simulink model "PlanarModelandControl"  (R2026a)
settings: Solver=FixedStepDiscrete  StopTime=StopTime  FixedStep=Ts  MaxStep=0  RelTol=1e-5  AbsTol=1e-3
PostLoadFcn:
  | Controles_Ativos = 1;
  | Vx_Ref = 0;
  | Vx_init = 0;
  | t_default = [0; 10000];
  | Brake_Cmd = [t_default, [0; 0]];
  | Accel_Cmd = [t_default, [0; 0]];
  | Steer_Cmd = [t_default, [0; 0]];
  | Flag_CruiseControl = 0;
  | Ts = 0.001;
  | load('TC_LUT.mat')
InitFcn:
  | % Carrega parâmetros gerais (Massa, Ganhos PID, Geometria)
  | run('Parameters.m');
  | 
  | % Carrega as Tabelas (Lookup Tables)
  | run('curva_steering.m');
  | run('curva_tq_motor.m');
  | load('TC_LUT.mat')
model workspace (MAT data in the model file):
  A = 1.22
  Ack = 1
  Cd = 1.1
  Cl = -2
  Cs = [19075.58 25992.8 31792.64 35898.02 39044.37]
  Fz0x = -811.9083
  Fz0y = -210.9467
  Fz_cs = [222.41 444.82 667.23 889.64 1112.05]
  IAY = 0
  IAx = 0
  IAy = 0
  Im = 0.00033
  It = 0.0094
  Iw = 0.1578
  Iw_ = 0.7
  Iz_car = 180
  K_50_understeer = 0.005184
  K_Stability = 1
  K_oversteer = -0.001
  Kappa_F = 0
  Kappa_R = 0
  Km_FM = 110000
  Km_Fm = 110000
  Km_RM = 120000
  Km_Rm = 120000
  KphiARB_F = 0
  KphiARB_R = 11000
  KphiF = 17054.5
  KphiR = 40034.8
  Kphim_F = 137500
  Kphim_R = 48600
  L = 1.53
  M = 300
  Mgt = 11.72
  P = 68.9476
  P0 = 55.4852
  R = 0.2032
  Rcurva = 20
  Re = 0.2
  SR = 3
  TV_On = 1
  Ts = 0.001
  W = 2940
  Wf = 308.034
  Wr = 339.426
  Ws = 2099.34
  YLL = 1.11022302463e-16
  cx1 = 1.3668
  cy1 = 1.839
  dpi = 0.242630467224
  dx1 = 2.1568
  dx2 = -0.065814
  dx3 = 0.0011254
  dy1 = 2.453
  dy2 = -0.11197
  dy3 = 10.2899
  ex1 = -0.19815
  ex2 = -0.62529
  ex3 = 1.3396
  ex4 = 0.0029464
  ey1 = 0.96085
  ey2 = -0.28662
  ey3 = 0.14389
  ey4 = 4.9274
  ey5 = -70.7165
  g = 9.81
  hcg = 0.252
  hcgs = 0.202
  hrc_f = 0.056
  hrc_r = 0.084
  hs_f = 0.222
  hs_r = 0.222
  hx1 = -0.003859
  hx2 = -0.000374
  hy1 = -0.000403
  hy2 = -0.000261
  kx1 = -36.9039
  kx2 = 22.3857
  kx3 = 0.61844
  ky1 = -83.2526
  ky2 = -5.565
  ky3 = 0.88491
  ky4 = 2.9994
  ky5 = -99.9393
  ky6 = -3.4705
  ky7 = -0.91452
  lf = 0.8415
  lr = 0.6885
  mass_FL = 67.5
  mass_FR = 67.5
  mass_RL = 82.5
  mass_RR = 82.5
  mi = 2
  ms = 240
  pty1 = 0.005744
  pty2 = 19.1334
  px1 = -0.039964
  px2 = -0.15015
  px3 = -0.32528
  px4 = -0.063273
  py1 = 0.38578
  py2 = 1.2048
  py3 = -0.14544
  py4 = 0.092886
  py5 = -0.82901
  r = 9
  rhoar = 1.22
  s_f = 1
  s_r = 0.9
  tf = 1.25
  tr = 1.25
  vx1 = -0.11922
  vx2 = 0.002205
  vy1 = 0.032907
  vy2 = 0.018068
  vy3 = -1.0429
  vy4 = 0.53712
  x_fl = 0.8415
  x_fr = 0.8415
  x_rl = 0.6885
  x_rr = 0.6885
  xs_f = 0.826
  xs_r = 0.704
  y_fl = 0.6
  y_fr = 0.6
  y_rl = 0.59
  y_rr = 0.59
values from neighbouring files (symbols this model uses):
  GG_mode = 0    [autocross.m]    (also in teste.mat)
  Gr = 11.72    [Parameters.m]    (also in teste.mat)
  K_variavel = [-0.001, 0, 0.0008, 0.0012, 0.0015]    [Parameters.m]    (also in teste.mat = [-0.001 0 0.0008 0.0012 0.0015])
  Ki_data = [283117.9493, 114831.615, 51596.0519, 30556.5344, 17525.221, 11773.8301, 10567.5001]*0.008    [Parameters.m]    (also in teste.mat = [2264.9435944 918.65292 412.7684152 244.4522752 140.201768 9)
  Kp_data = [2877.5702, 2303.9999, 1972.0835, 1556.8775, 1258.2451, 1022.7085, 982.7861, ]*5    [Parameters.m]    (also in teste.mat = [14387.851 11519.9995 9860.4175 7784.3875 6291.2255 5113.542)
  Mz_maxF = [0 66.3 132.6 198.9 265.2 330.23 396.52 462.83 529.112 595.42 661.73 728.03 794.33 860.62 926.92 993.23 1058.25 1124.55 1190.85 1257.15 1323.45 1389.75 1456.05 1522.35 1588.65 1654.95]    [Parameters.m]    (also in teste.mat = [0 66.3 132.6 198.9 265.2 330.23 396.52 462.83 529.112 595.4)
  Mz_minF = [0 -66.3 -132.6 -198.9 -265.2 -330.23 -396.52 -462.83 -529.112 -595.42 -661.73 -728.03 -794.33 -860.62 -926.92 -993.23 -1058.25 -1124.55 -1190.85 -1257.15 -1323.45 -1389.75 -1456.05 -1522.35 -1588.65 -1654.95]    [Parameters.m]    (also in teste.mat = [0 -66.3 -132.6 -198.9 -265.2 -330.23 -396.52 -462.83 -529.1)
  pedal = [0 4 8 12 16 20 24 28 32 36 40 44 48 52 56 60 64 68 72 76 80 84 88 92 96 100]    [Parameters.m]    (also in teste.mat = [0 4 8 12 16 20 24 28 32 36 40 44 ... (26 elements, 1x26)])
  rpm_355_novo = [0 10050 10100 10140 10170 10210 10250 10280 10320 10360         10390 10420 10460 10620 10770 10920 11050 11170 11280         11380 11470 11550 11625 11680 11730 11775 11790 11800]    [curva_tq_motor.m]    (also in teste.mat = [0 10050 10100 10140 10170 10210 10250 10280 10320 10360 103)
  Steering_inner = [-29.018 ... (first row only)    [curva_steering.m]    (also in teste.mat = [-29.018 -25.595 -22.357 -19.265 -16.292 -13.415 -10.619 -7.)
  Steering_outer = [-25.111 ... (first row only)    [curva_steering.m]    (also in teste.mat = [-25.111 -22.589 -20.082 -17.585 -15.094 -12.604 -10.11 -7.6)
  steering_vol = [-105 ... (first row only)    [curva_steering.m]    (also in teste.mat = [-105 -94.5 -84 -73.5 -63 -52.5 -42 -31.5 -21 -10.5 0 10.5 .)
  torque_355_novo = [16.2 16.2 16.0 15.8 15.6 15.4 15.2 15.0 14.8 14.6            14.4 14.2 14.0 13.0 12.0 11.0 10.0 9.0 8.0            7.0 6.0 5.0 4.0 3.0 2.0 1.0 0.5 0.0]    [curva_tq_motor.m]    (also in teste.mat = [16.2 16.2 16 15.8 15.6 15.4 15.2 15 14.8 14.6 14.4 14.2 ...)
  Vx = [0, 12.5, 23.6, 30, 35]    [Parameters.m]    (also in teste.mat = [0 12.5 23.6 30 35])
  Vx_axis = [5, 10, 15, 20, 25, 30, 35]    [Parameters.m]    (also in teste.mat = [5 10 15 20 25 30 35])
undefined symbols (not defined in the file or in its folder): DiscIntNeverNeededParam, magnitude
libraries: simulink x5, fuzblock x4, sl_discontinuities x3, simulink_extras x3, slpidlib x1
(part 4 of 4)

== system PlanarModelandControl/Planta/Dinamica do Veículo/Pacejka/Pacejka Long FL /Dx ==
  Dfz : Inport
  Bus Element In1 : Inport  Port=2  PortName=Fz
  Constant : Constant  Value=dx1  SampleTime=0.01
  Gain : Gain  Gain=dx2
  Gain1 : Gain  Gain=1+px1*dpi+px2*dpi^2
  Gain2 : Gain  Gain=1-dx3*IAx^2
  Gain3 : Gain  Gain=-1
  Product : Product
  Sum : Sum  Inputs=|++
  Dx : Outport
  wires:
  Constant/1 -> Sum/1
  Gain/1 -> Sum/2
  Dfz/1 -> Gain/1
  Sum/1 -> Gain1/1
  Product/1 -> Dx/1
  Bus Element In1/1 -> Gain3/1
  Gain1/1 -> Gain2/1
  Gain2/1 -> Product/1
  Gain3/1 -> Product/2

== system PlanarModelandControl/Planta/Dinamica do Veículo/Pacejka/Pacejka Long FL /Ex ==
  Dfz : Inport
  SL : Inport  Port=2
  Abs : Abs  SaturateOnIntegerOverflow=off
  Constant : Constant  Value=ex1  SampleTime=0.01
  Constant2 : Constant  SampleTime=0.01
  Gain : Gain  Gain=ex3
  Gain1 : Gain  Gain=ex4
  Gain2 : Gain  Gain=ex2
  Min : MinMax  Inputs=2
  Product : Product
  Product1 : Product
  Sign : Signum
  Square : Math  Operator=square
  Sum : Sum  Inputs=|+++
  Sum1 : Sum  Inputs=|+-
  Ex : Outport
  wires:
  Constant/1 -> Sum/1
  Gain2/1 -> Sum/2
  Square/1 -> Gain/1
  Gain/1 -> Sum/3
  Sum/1 -> Product/1
  Sum1/1 -> Product/2
  Product/1 -> Min/1
  Sign/1 -> Product1/2
  Abs/1 -> Product1/1
  Constant2/1 -> Min/2, Sum1/1, Abs/1
  Gain1/1 -> Sum1/2
  Product1/1 -> Gain1/1
  Dfz/1 -> Square/1, Gain2/1
  SL/1 -> Sign/1
  Min/1 -> Ex/1

== system PlanarModelandControl/Planta/Dinamica do Veículo/Pacejka/Pacejka Long FL /Kx ==
  Bus Element In1 : Inport  PortName=Fz
  Dfz : Inport  Port=2
  Constant : Constant  Value=kx1  SampleTime=0.01
  Exp : Math
  Gain : Gain  Gain=kx3
  Gain1 : Gain  Gain=kx2
  Gain2 : Gain  Gain=-1
  Gain3 : Gain  Gain=1+px3*dpi+px4*dpi^2
  Product : Product
  Product1 : Product
  Sum : Sum  Inputs=|++
  Kx : Outport
  wires:
  Bus Element In1/1 -> Gain2/1
  Product/1 -> Product1/1
  Exp/1 -> Product1/2
  Gain1/1 -> Sum/2
  Sum/1 -> Product/2
  Product1/1 -> Gain3/1
  Constant/1 -> Sum/1
  Gain/1 -> Exp/1
  Dfz/1 -> Gain1/1, Gain/1
  Gain2/1 -> Product/1
  Gain3/1 -> Kx/1

== system PlanarModelandControl/Planta/Dinamica do Veículo/Pacejka/Pacejka Long FL /Slip ==
  Dfz : Inport
  SL : Inport  Port=2
  Constant : Constant  Value=hx1
  Gain : Gain  Gain=hx2
  Sum : Sum  Inputs=|++
  Sum1 : Sum  Inputs=|++
  "Slx " : Outport
  wires:
  Dfz/1 -> Gain/1
  Constant/1 -> Sum1/1
  Gain/1 -> Sum1/2
  SL/1 -> Sum/1
  Sum/1 -> "Slx "/1
  Sum1/1 -> Sum/2

== system PlanarModelandControl/Planta/Dinamica do Veículo/Pacejka/Pacejka Long FL /Svx ==
  Dfz : Inport
  Bus Element In1 : Inport  Port=2  PortName=Fz
  Constant : Constant  Value=vx1  SampleTime=0.01
  Gain : Gain  Gain=vx2
  Gain1 : Gain  Gain=-1
  Product : Product
  Sum : Sum  Inputs=|++
  Svx : Outport
  wires:
  Bus Element In1/1 -> Gain1/1
  Sum/1 -> Product/2
  Gain/1 -> Sum/2
  Dfz/1 -> Gain/1
  Constant/1 -> Sum/1
  Product/1 -> Svx/1
  Gain1/1 -> Product/1

== system PlanarModelandControl/Planta/Dinamica do Veículo/Pacejka/Pacejka Long FL /dfz ==
  Bus Element In1 : Inport  PortName=Fz
  Constant : Constant  Value=Fz0x
  Divide : Product  Inputs=*/
  Gain : Gain  Gain=-1
  Sum : Sum  Inputs=|+-
  Dfz : Outport
  wires:
  Constant/1 -> Sum/2, Divide/2
  Sum/1 -> Divide/1
  Divide/1 -> Dfz/1
  Bus Element In1/1 -> Gain/1
  Gain/1 -> Sum/1

== system PlanarModelandControl/Planta/Dinamica do Veículo/Pacejka/Pacejka Long FR/Bx ==
  Kx : Inport
  Dx : Inport  Port=2
  Divide : Product  Inputs=*/
  Gain : Gain  Gain=cx1
  Bx : Outport
  wires:
  Kx/1 -> Divide/1
  Dx/1 -> Gain/1
  Gain/1 -> Divide/2
  Divide/1 -> Bx/1

== system PlanarModelandControl/Planta/Dinamica do Veículo/Pacejka/Pacejka Long FR/Dx ==
  Dfz : Inport
  Bus Element In1 : Inport  Port=2  PortName=Fz
  Constant : Constant  Value=dx1  SampleTime=0.01
  Gain : Gain  Gain=dx2
  Gain1 : Gain  Gain=1+px1*dpi+px2*dpi^2
  Gain2 : Gain  Gain=1-dx3*IAx^2
  Gain3 : Gain  Gain=-1
  Product : Product
  Sum : Sum  Inputs=|++
  Dx : Outport
  wires:
  Constant/1 -> Sum/1
  Gain/1 -> Sum/2
  Dfz/1 -> Gain/1
  Sum/1 -> Gain1/1
  Product/1 -> Dx/1
  Bus Element In1/1 -> Gain3/1
  Gain1/1 -> Gain2/1
  Gain2/1 -> Product/1
  Gain3/1 -> Product/2

== system PlanarModelandControl/Planta/Dinamica do Veículo/Pacejka/Pacejka Long FR/Ex ==
  Dfz : Inport
  SL : Inport  Port=2
  Abs : Abs  SaturateOnIntegerOverflow=off
  Constant : Constant  Value=ex1  SampleTime=0.01
  Constant2 : Constant  SampleTime=0.01
  Gain : Gain  Gain=ex3
  Gain1 : Gain  Gain=ex4
  Gain2 : Gain  Gain=ex2
  Min : MinMax  Inputs=2
  Product : Product
  Product1 : Product
  Sign : Signum
  Square : Math  Operator=square
  Sum : Sum  Inputs=|+++
  Sum1 : Sum  Inputs=|+-
  Ex : Outport
  wires:
  Constant/1 -> Sum/1
  Gain2/1 -> Sum/2
  Square/1 -> Gain/1
  Gain/1 -> Sum/3
  Sum/1 -> Product/1
  Sum1/1 -> Product/2
  Product/1 -> Min/1
  Sign/1 -> Product1/2
  Abs/1 -> Product1/1
  Constant2/1 -> Min/2, Sum1/1, Abs/1
  Gain1/1 -> Sum1/2
  Product1/1 -> Gain1/1
  Dfz/1 -> Square/1, Gain2/1
  SL/1 -> Sign/1
  Min/1 -> Ex/1

== system PlanarModelandControl/Planta/Dinamica do Veículo/Pacejka/Pacejka Long FR/Kx ==
  Bus Element In1 : Inport  PortName=Fz
  Dfz : Inport  Port=2
  Constant : Constant  Value=kx1  SampleTime=0.01
  Exp : Math
  Gain : Gain  Gain=kx3
  Gain1 : Gain  Gain=kx2
  Gain2 : Gain  Gain=-1
  Gain3 : Gain  Gain=1+px3*dpi+px4*dpi^2
  Product : Product
  Product1 : Product
  Sum : Sum  Inputs=|++
  Kx : Outport
  wires:
  Bus Element In1/1 -> Gain2/1
  Product/1 -> Product1/1
  Exp/1 -> Product1/2
  Gain1/1 -> Sum/2
  Sum/1 -> Product/2
  Product1/1 -> Gain3/1
  Constant/1 -> Sum/1
  Gain/1 -> Exp/1
  Dfz/1 -> Gain1/1, Gain/1
  Gain2/1 -> Product/1
  Gain3/1 -> Kx/1

== system PlanarModelandControl/Planta/Dinamica do Veículo/Pacejka/Pacejka Long FR/Slip ==
  Dfz : Inport
  SL : Inport  Port=2
  Constant : Constant  Value=hx1
  Gain : Gain  Gain=hx2
  Sum : Sum  Inputs=|++
  Sum1 : Sum  Inputs=|++
  "Slx " : Outport
  wires:
  Dfz/1 -> Gain/1
  Constant/1 -> Sum1/1
  Gain/1 -> Sum1/2
  SL/1 -> Sum/1
  Sum/1 -> "Slx "/1
  Sum1/1 -> Sum/2

== system PlanarModelandControl/Planta/Dinamica do Veículo/Pacejka/Pacejka Long FR/Svx ==
  Dfz : Inport
  Bus Element In1 : Inport  Port=2  PortName=Fz
  Constant : Constant  Value=vx1  SampleTime=0.01
  Gain : Gain  Gain=vx2
  Gain1 : Gain  Gain=-1
  Product : Product
  Sum : Sum  Inputs=|++
  Svx : Outport
  wires:
  Bus Element In1/1 -> Gain1/1
  Sum/1 -> Product/2
  Gain/1 -> Sum/2
  Dfz/1 -> Gain/1
  Constant/1 -> Sum/1
  Product/1 -> Svx/1
  Gain1/1 -> Product/1

== system PlanarModelandControl/Planta/Dinamica do Veículo/Pacejka/Pacejka Long FR/dfz ==
  Bus Element In1 : Inport  PortName=Fz
  Constant : Constant  Value=Fz0x
  Divide : Product  Inputs=*/
  Gain : Gain  Gain=-1
  Sum : Sum  Inputs=|+-
  Dfz : Outport
  wires:
  Constant/1 -> Sum/2, Divide/2
  Sum/1 -> Divide/1
  Divide/1 -> Dfz/1
  Bus Element In1/1 -> Gain/1
  Gain/1 -> Sum/1

== system PlanarModelandControl/Planta/Dinamica do Veículo/Pacejka/Pacejka Long RL/Bx ==
  Kx : Inport
  Dx : Inport  Port=2
  Divide : Product  Inputs=*/
  Gain : Gain  Gain=cx1
  Bx : Outport
  wires:
  Kx/1 -> Divide/1
  Dx/1 -> Gain/1
  Gain/1 -> Divide/2
  Divide/1 -> Bx/1

== system PlanarModelandControl/Planta/Dinamica do Veículo/Pacejka/Pacejka Long RL/Dx ==
  Dfz : Inport
  Bus Element In1 : Inport  Port=2  PortName=Fz
  Constant : Constant  Value=dx1  SampleTime=0.01
  Gain : Gain  Gain=dx2
  Gain1 : Gain  Gain=1+px1*dpi+px2*dpi^2
  Gain2 : Gain  Gain=1-dx3*IAx^2
  Gain3 : Gain  Gain=-1
  Product : Product
  Sum : Sum  Inputs=|++
  Dx : Outport
  wires:
  Constant/1 -> Sum/1
  Gain/1 -> Sum/2
  Dfz/1 -> Gain/1
  Sum/1 -> Gain1/1
  Product/1 -> Dx/1
  Bus Element In1/1 -> Gain3/1
  Gain1/1 -> Gain2/1
  Gain2/1 -> Product/1
  Gain3/1 -> Product/2

== system PlanarModelandControl/Planta/Dinamica do Veículo/Pacejka/Pacejka Long RL/Ex ==
  Dfz : Inport
  SL : Inport  Port=2
  Abs : Abs  SaturateOnIntegerOverflow=off
  Constant : Constant  Value=ex1  SampleTime=0.01
  Constant2 : Constant  SampleTime=0.01
  Gain : Gain  Gain=ex3
  Gain1 : Gain  Gain=ex4
  Gain2 : Gain  Gain=ex2
  Min : MinMax  Inputs=2
  Product : Product
  Product1 : Product
  Sign : Signum
  Square : Math  Operator=square
  Sum : Sum  Inputs=|+++
  Sum1 : Sum  Inputs=|+-
  Ex : Outport
  wires:
  Constant/1 -> Sum/1
  Gain2/1 -> Sum/2
  Square/1 -> Gain/1
  Gain/1 -> Sum/3
  Sum/1 -> Product/1
  Sum1/1 -> Product/2
  Product/1 -> Min/1
  Sign/1 -> Product1/2
  Abs/1 -> Product1/1
  Constant2/1 -> Min/2, Sum1/1, Abs/1
  Gain1/1 -> Sum1/2
  Product1/1 -> Gain1/1
  Dfz/1 -> Square/1, Gain2/1
  SL/1 -> Sign/1
  Min/1 -> Ex/1

== system PlanarModelandControl/Planta/Dinamica do Veículo/Pacejka/Pacejka Long RL/Kx ==
  Bus Element In1 : Inport  PortName=Fz
  Dfz : Inport  Port=2
  Constant : Constant  Value=kx1  SampleTime=0.01
  Exp : Math
  Gain : Gain  Gain=kx3
  Gain1 : Gain  Gain=kx2
  Gain2 : Gain  Gain=-1
  Gain3 : Gain  Gain=1+px3*dpi+px4*dpi^2
  Product : Product
  Product1 : Product
  Sum : Sum  Inputs=|++
  Kx : Outport
  wires:
  Bus Element In1/1 -> Gain2/1
  Product/1 -> Product1/1
  Exp/1 -> Product1/2
  Gain1/1 -> Sum/2
  Sum/1 -> Product/2
  Product1/1 -> Gain3/1
  Constant/1 -> Sum/1
  Gain/1 -> Exp/1
  Dfz/1 -> Gain1/1, Gain/1
  Gain2/1 -> Product/1
  Gain3/1 -> Kx/1

== system PlanarModelandControl/Planta/Dinamica do Veículo/Pacejka/Pacejka Long RL/Slip ==
  Dfz : Inport
  SL : Inport  Port=2
  Constant : Constant  Value=hx1
  Gain : Gain  Gain=hx2
  Sum : Sum  Inputs=|++
  Sum1 : Sum  Inputs=|++
  "Slx " : Outport
  wires:
  Dfz/1 -> Gain/1
  Constant/1 -> Sum1/1
  Gain/1 -> Sum1/2
  SL/1 -> Sum/1
  Sum/1 -> "Slx "/1
  Sum1/1 -> Sum/2

== system PlanarModelandControl/Planta/Dinamica do Veículo/Pacejka/Pacejka Long RL/Svx ==
  Dfz : Inport
  Bus Element In1 : Inport  Port=2  PortName=Fz
  Constant : Constant  Value=vx1  SampleTime=0.01
  Gain : Gain  Gain=vx2
  Gain1 : Gain  Gain=-1
  Product : Product
  Sum : Sum  Inputs=|++
  Svx : Outport
  wires:
  Bus Element In1/1 -> Gain1/1
  Sum/1 -> Product/2
  Gain/1 -> Sum/2
  Dfz/1 -> Gain/1
  Constant/1 -> Sum/1
  Product/1 -> Svx/1
  Gain1/1 -> Product/1

== system PlanarModelandControl/Planta/Dinamica do Veículo/Pacejka/Pacejka Long RL/dfz ==
  Bus Element In1 : Inport  PortName=Fz
  Constant : Constant  Value=Fz0x
  Divide : Product  Inputs=*/
  Gain : Gain  Gain=-1
  Sum : Sum  Inputs=|+-
  Dfz : Outport
  wires:
  Constant/1 -> Sum/2, Divide/2
  Sum/1 -> Divide/1
  Divide/1 -> Dfz/1
  Bus Element In1/1 -> Gain/1
  Gain/1 -> Sum/1

== system PlanarModelandControl/Planta/Dinamica do Veículo/Pacejka/Pacejka Long RR/Bx ==
  Kx : Inport
  Dx : Inport  Port=2
  Divide : Product  Inputs=*/
  Gain : Gain  Gain=cx1
  Bx : Outport
  wires:
  Kx/1 -> Divide/1
  Dx/1 -> Gain/1
  Gain/1 -> Divide/2
  Divide/1 -> Bx/1

== system PlanarModelandControl/Planta/Dinamica do Veículo/Pacejka/Pacejka Long RR/Dx ==
  Dfz : Inport
  Bus Element In1 : Inport  Port=2  PortName=Fz
  Constant : Constant  Value=dx1  SampleTime=0.01
  Gain : Gain  Gain=dx2
  Gain1 : Gain  Gain=1+px1*dpi+px2*dpi^2
  Gain2 : Gain  Gain=1-dx3*IAx^2
  Gain3 : Gain  Gain=-1
  Product : Product
  Sum : Sum  Inputs=|++
  Dx : Outport
  wires:
  Constant/1 -> Sum/1
  Gain/1 -> Sum/2
  Dfz/1 -> Gain/1
  Sum/1 -> Gain1/1
  Product/1 -> Dx/1
  Bus Element In1/1 -> Gain3/1
  Gain1/1 -> Gain2/1
  Gain2/1 -> Product/1
  Gain3/1 -> Product/2

== system PlanarModelandControl/Planta/Dinamica do Veículo/Pacejka/Pacejka Long RR/Ex ==
  Dfz : Inport
  SL : Inport  Port=2
  Abs : Abs  SaturateOnIntegerOverflow=off
  Constant : Constant  Value=ex1  SampleTime=0.01
  Constant2 : Constant  SampleTime=0.01
  Gain : Gain  Gain=ex3
  Gain1 : Gain  Gain=ex4
  Gain2 : Gain  Gain=ex2
  Min : MinMax  Inputs=2
  Product : Product
  Product1 : Product
  Sign : Signum
  Square : Math  Operator=square
  Sum : Sum  Inputs=|+++
  Sum1 : Sum  Inputs=|+-
  Ex : Outport
  wires:
  Constant/1 -> Sum/1
  Gain2/1 -> Sum/2
  Square/1 -> Gain/1
  Gain/1 -> Sum/3
  Sum/1 -> Product/1
  Sum1/1 -> Product/2
  Product/1 -> Min/1
  Sign/1 -> Product1/2
  Abs/1 -> Product1/1
  Constant2/1 -> Min/2, Sum1/1, Abs/1
  Gain1/1 -> Sum1/2
  Product1/1 -> Gain1/1
  Dfz/1 -> Square/1, Gain2/1
  SL/1 -> Sign/1
  Min/1 -> Ex/1

== system PlanarModelandControl/Planta/Dinamica do Veículo/Pacejka/Pacejka Long RR/Kx ==
  Bus Element In1 : Inport  PortName=Fz
  Dfz : Inport  Port=2
  Constant : Constant  Value=kx1  SampleTime=0.01
  Exp : Math
  Gain : Gain  Gain=kx3
  Gain1 : Gain  Gain=kx2
  Gain2 : Gain  Gain=-1
  Gain3 : Gain  Gain=1+px3*dpi+px4*dpi^2
  Product : Product
  Product1 : Product
  Sum : Sum  Inputs=|++
  Kx : Outport
  wires:
  Bus Element In1/1 -> Gain2/1
  Product/1 -> Product1/1
  Exp/1 -> Product1/2
  Gain1/1 -> Sum/2
  Sum/1 -> Product/2
  Product1/1 -> Gain3/1
  Constant/1 -> Sum/1
  Gain/1 -> Exp/1
  Dfz/1 -> Gain1/1, Gain/1
  Gain2/1 -> Product/1
  Gain3/1 -> Kx/1

== system PlanarModelandControl/Planta/Dinamica do Veículo/Pacejka/Pacejka Long RR/Slip ==
  Dfz : Inport
  SL : Inport  Port=2
  Constant : Constant  Value=hx1
  Gain : Gain  Gain=hx2
  Sum : Sum  Inputs=|++
  Sum1 : Sum  Inputs=|++
  "Slx " : Outport
  wires:
  Dfz/1 -> Gain/1
  Constant/1 -> Sum1/1
  Gain/1 -> Sum1/2
  SL/1 -> Sum/1
  Sum/1 -> "Slx "/1
  Sum1/1 -> Sum/2

== system PlanarModelandControl/Planta/Dinamica do Veículo/Pacejka/Pacejka Long RR/Svx ==
  Dfz : Inport
  Bus Element In1 : Inport  Port=2  PortName=Fz
  Constant : Constant  Value=vx1  SampleTime=0.01
  Gain : Gain  Gain=vx2
  Gain1 : Gain  Gain=-1
  Product : Product
  Sum : Sum  Inputs=|++
  Svx : Outport
  wires:
  Bus Element In1/1 -> Gain1/1
  Sum/1 -> Product/2
  Gain/1 -> Sum/2
  Dfz/1 -> Gain/1
  Constant/1 -> Sum/1
  Product/1 -> Svx/1
  Gain1/1 -> Product/1

== system PlanarModelandControl/Planta/Dinamica do Veículo/Pacejka/Pacejka Long RR/dfz ==
  Bus Element In1 : Inport  PortName=Fz
  Constant : Constant  Value=Fz0x
  Divide : Product  Inputs=*/
  Gain : Gain  Gain=-1
  Sum : Sum  Inputs=|+-
  Dfz : Outport
  wires:
  Constant/1 -> Sum/2, Divide/2
  Sum/1 -> Divide/1
  Divide/1 -> Dfz/1
  Bus Element In1/1 -> Gain/1
  Gain/1 -> Sum/1

== matlab function PlanarModelandControl/Planta/Dinamica do Veículo/Pacejka/Pacejka Long RL/MATLAB Function ==
  data: Slx input; Fx output size=1; Dx input; Cx input; Bx input; Ex input; SVx input
function Fx = ComputeFx(Slx, Dx, Cx, Bx, Ex, SVx)

Fx = Dx .* sin( Cx .* atan( Bx .* Slx- Ex * ( Bx * Slx - atan(Bx .* Slx) ) ) ) + SVx;

== matlab function PlanarModelandControl/Planta/Dinamica do Veículo/Pacejka/Pacejka Long RR/MATLAB Function ==
  data: Slx input; Fx output size=1; Dx input; Cx input; Bx input; Ex input; SVx input
function Fx = ComputeFx(Slx, Dx, Cx, Bx, Ex, SVx)

Fx = Dx .* sin( Cx .* atan( Bx .* Slx- Ex * ( Bx * Slx - atan(Bx .* Slx) ) ) ) + SVx;

== matlab function PlanarModelandControl/Planta/Dinamica do Veículo/Load Transfer/Total Normal Forces/MATLAB Function4 ==
  data: u input; y output
function y = fcn(u)

if u <= 0
    y = 0;
else
    y = u;
end

== matlab function PlanarModelandControl/Planta/Dinamica do Veículo/Load Transfer/Total Normal Forces/MATLAB Function5 ==
  data: u input; y output
function y = fcn(u)

if u <= 0
    y = 0;
else
    y = u;
end

== matlab function PlanarModelandControl/Planta/Dinamica do Veículo/Pacejka/Pacejka Lat FL1/MATLAB Function ==
  data: Fz input; Dy input; Cy input; By input; Ey input; SVy input; SAy input; Fy output size=1; SA input
function Fy = computeFy(Fz, Dy, Cy, By, Ey, SVy, SAy, SA)
   if SA == 0 || Fz <= 0
       Fy = 0;
   else
       Fy = Dy .* sin( Cy .* atan( By .* SAy - Ey .* ( By .* SAy - atan(By .* SAy) ) ) ) + SVy;
   end
end

== matlab function PlanarModelandControl/Planta/Dinamica do Veículo/Pacejka/Pacejka Lat FL2/MATLAB Function ==
  data: Fz input; Dy input; Cy input; By input; Ey input; SVy input; SAy input; Fy output size=1; SA input
function Fy = computeFy(Fz, Dy, Cy, By, Ey, SVy, SAy, SA)
   if SA == 0 || Fz <= 0
       Fy = 0;
   else
       Fy = Dy .* sin( Cy .* atan( By .* SAy - Ey .* ( By .* SAy - atan(By .* SAy) ) ) ) + SVy;
   end
end

== matlab function PlanarModelandControl/Planta/Dinamica do Veículo/Pacejka/Pacejka Lat FL3/MATLAB Function ==
  data: Fz input; Dy input; Cy input; By input; Ey input; SVy input; SAy input; Fy output size=1; SA input
function Fy = computeFy(Fz, Dy, Cy, By, Ey, SVy, SAy, SA)
   if SA == 0 || Fz <= 0
       Fy = 0;
   else
       Fy = Dy .* sin( Cy .* atan( By .* SAy - Ey .* ( By .* SAy - atan(By .* SAy) ) ) ) + SVy;
   end
end

== matlab function PlanarModelandControl/Planta/Dinamica do Veículo/Pacejka/Pacejka Lat FL4/MATLAB Function ==
  data: Fz input; Dy input; Cy input; By input; Ey input; SVy input; SAy input; Fy output size=1; SA input
function Fy = computeFy(Fz, Dy, Cy, By, Ey, SVy, SAy, SA)
   if SA == 0 || Fz <= 0
       Fy = 0;
   else
       Fy = Dy .* sin( Cy .* atan( By .* SAy - Ey .* ( By .* SAy - atan(By .* SAy) ) ) ) + SVy;
   end
end

== matlab function PlanarModelandControl/Planta/Dinamica do Veículo/Pacejka/Pacejka Long FR/MATLAB Function ==
  data: Slx input; Fx output size=1; Dx input; Cx input; Bx input; Ex input; SVx input
function Fx = ComputeFx(Slx, Dx, Cx, Bx, Ex, SVx)

Fx = Dx .* sin( Cx .* atan( Bx .* Slx- Ex * ( Bx * Slx - atan(Bx .* Slx) ) ) ) + SVx;

== matlab function PlanarModelandControl/Planta/Movimento do Veiculo/Ref Inercial - Velocidade/MATLAB Function ==
  data: Vx input; y output; Vy input
function y = fcn(Vx, Vy)

if Vx == 0 || Vy == 0
    y = 0;
else 
    y = Vy/Vx;
end

== matlab function PlanarModelandControl/Planta/Dinamica do Veículo/Pacejka/Pacejka Long FL /MATLAB Function ==
  data: Slx input; Fx output size=1; Dx input; Cx input; Bx input; Ex input; SVx input
function Fx = ComputeFx(Slx, Dx, Cx, Bx, Ex, SVx)

Fx = Dx .* sin( Cx .* atan( Bx .* Slx- Ex * ( Bx * Slx - atan(Bx .* Slx) ) ) ) + SVx;
